simulink model "v13_aa_rii_16r128dr_ver104"  (Simulink 7.9)
settings: Solver=FixedStepDiscrete  SolverMode=SingleTasking  StartTime=0.0  StopTime=32768  RelTol=1e-3
undefined symbols (not defined in the file or in its folder): sfp
libraries: xbsIndex_r4 x19795, xps_library x54, casper_library_misc x36, dspsigops x32
(part 1 of 212)
settings Model: Creator=jayanth  LastModifiedBy=mwagner
settings DebuggingCC: FcnCallInpInsideContextMsg=Use local settings
settings GRTTargetCC: CombineOutputUpdateFcns=off  GRTInterface=on

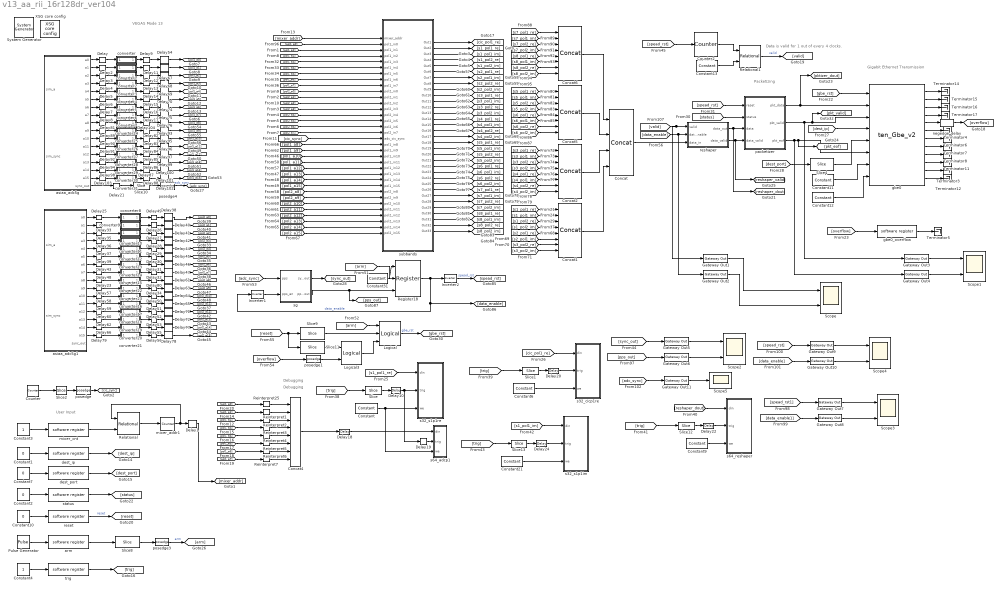
== system v13_aa_rii_16r128dr_ver104 ==
  note: Gigabit Ethernet Transmission
  note: Debugging
  note: Debugging
  note: Packetizing
  note: Data is valid for 1 out of every 4 clocks.
  note: VEGAS Mode 13
  note: User Input
  " System Generator" : xbsIndex_r4/ System Generator  Tag=genX  xilinxfamily=virtex6  part=xc6vsx475t  speed=-1  package=ff1759  synthesis_tool=XST  clock_wrapper=Clock Enables  directory=./sn/sysgen  proj_type=Project Navigator  Synth_file=XST Defaults  Impl_file=ISE Defaults  testbench=off  simulink_period=1  sysclk_period=5.3191  dcm_input_clock_period=100  incr_netlist=off  trim_vbits=Everywhere in SubSystem  dbl_ovrd=According to Block Masks  core_generation=According to Block Masks  run_coregen=off  deprecated_control=off  eval_field=0  block_type=sysgen
  Concat : xbsIndex_r4/Concat  num_inputs=4  dbl_ovrd=off  block_type=concat
  Concat{1..2,4..6} (5 alike) : xbsIndex_r4/Concat  num_inputs=8  dbl_ovrd=off  block_type=concat
  Constant{,6,9,21} (4 alike) : xbsIndex_r4/Constant  const=1  gui_display_data_type=Boolean  arith_type=Boolean  n_bits=16  bin_pt=14  preci_type=Single  exp_width=8  frac_width=24  explicit_period=off  period=1  equ=P=C  opselect=C  inp2=PCIN>>17  opr=+  inp1=P  carry=CIN  dbl_ovrd=off  block_type=constant
  Constant{1..2,7,10} (4 alike) : Constant  Value=0
  Constant11 : xbsIndex_r4/Constant  const=1  gui_display_data_type=Boolean  arith_type=Boolean  n_bits=16  bin_pt=14  preci_type=Single  exp_width=8  frac_width=24  explicit_period=on  period=1  equ=P=C  opselect=C  inp2=PCIN>>17  opr=+  inp1=P  carry=CIN  dbl_ovrd=off  block_type=constant
  Constant12 : xbsIndex_r4/Constant  const=0  gui_display_data_type=Boolean  arith_type=Boolean  n_bits=16  bin_pt=14  preci_type=Single  exp_width=8  frac_width=24  explicit_period=on  period=1  equ=P=C  opselect=C  inp2=PCIN>>17  opr=+  inp1=P  carry=CIN  dbl_ovrd=off  block_type=constant
  Constant13 : xbsIndex_r4/Constant  const=7  gui_display_data_type=Fixed-point  arith_type=Unsigned  n_bits=3  bin_pt=0  preci_type=Single  exp_width=8  frac_width=24  explicit_period=on  period=1  equ=P=C  opselect=C  inp2=PCIN>>17  opr=+  inp1=P  carry=CIN  dbl_ovrd=off  block_type=constant
  Constant3 : Constant
  Constant31 : xbsIndex_r4/Constant  const=1  gui_display_data_type=Boolean  arith_type=Boolean  n_bits=16  bin_pt=0  preci_type=Single  exp_width=8  frac_width=24  explicit_period=on  period=1  equ=P=C  opselect=C  inp2=PCIN>>17  opr=+  inp1=P  carry=CIN  dbl_ovrd=off  block_type=constant
      settings: block_version=11.4
  Constant4 : Constant
  Counter : xbsIndex_r4/Counter  cnt_type=Free Running  cnt_to=Inf  operation=Up  start_count=0  cnt_by_val=1  arith_type=Unsigned  n_bits=16  bin_pt=0  load_pin=off  rst=off  en=off  explicit_period=on  period=1  dbl_ovrd=off  use_behavioral_HDL=off  implementation=Fabric  block_type=counter
  Counter2 : xbsIndex_r4/Counter  cnt_type=Free Running  cnt_to=Inf  operation=Up  start_count=2  cnt_by_val=1  arith_type=Unsigned  n_bits=3  bin_pt=0  load_pin=off  rst=on  en=off  explicit_period=on  period=1  dbl_ovrd=off  use_behavioral_HDL=off  implementation=Fabric  block_type=counter
      settings: block_version=11.4
  Delay{,2..8,21,23,25,33,35..37,39,43,48,57..60,62,66,79,81,89..92,94,98,103} (33 alike) : xbsIndex_r4/Delay  rst=off  infoeditControl=Selection of Reset will increase slice count due to use of real FFs and instead of SRLs  en=off  latency=3  dbl_ovrd=off  reg_retiming=off  block_type=delay
  Delay1 : xbsIndex_r4/Delay  rst=off  infoeditControl=Selection of Reset will increase slice count due to use of real FFs and instead of SRLs  en=off  latency=2  dbl_ovrd=off  reg_retiming=on  block_type=delay
      settings: block_version=11.4
  Delay{10,18..20,22,24} (6 alike) : xbsIndex_r4/Delay  rst=off  infoeditControl=Selection of Reset will increase slice count due to use of real FFs and instead of SRLs  en=off  latency=20  dbl_ovrd=off  reg_retiming=off  block_type=delay
  Delay{38,40..42,44..47,54,61,63..65,67..72,74..78,93,95..97,99..102} (32 alike) : xbsIndex_r4/Delay  rst=off  infoeditControl=Selection of Reset will increase slice count due to use of real FFs and instead of SRLs  en=off  latency=3  dbl_ovrd=off  reg_retiming=on  block_type=delay
      settings: block_version=11.4
  Delay{9,11..17,26..32,34,49..53,55..56,80,82..88,104} (32 alike) : xbsIndex_r4/Delay  rst=off  infoeditControl=Selection of Reset will increase slice count due to use of real FFs and instead of SRLs  en=off  latency=8  dbl_ovrd=off  reg_retiming=off  block_type=delay
  Gateway Out{1..11} (11 alike) : xbsIndex_r4/Gateway Out  inherit_from_input=off  hdl_port=on  timing_constraint=None  locs_specified=off  LOCs={}  UseAsDAC=off  DACChannel='1'  block_type=gatewayout
      settings: infoedit="Gateway out block.  Converts Xilinx fixed point or floating-point type inputs into ouputs of type Simulink integer, double, or fixed point.<br><br>Hardware notes:  In hardware these blocks become top level output ports or are discarded, depending on how they are configured."
  Inverter1 : xbsIndex_r4/Inverter  en=off  latency=0  dbl_ovrd=off  block_type=inv
      settings: block_version=11.4
  Inverter2 : xbsIndex_r4/Inverter  en=off  latency=0  dbl_ovrd=off  block_type=inv
      settings: block_version=11.4
  Logical : xbsIndex_r4/Logical  logical_function=OR  inputs=3  en=off  latency=0  precision=Full  arith_type=Unsigned  n_bits=16  bin_pt=0  align_bp=on  dbl_ovrd=off  block_type=logical
  Logical3 : xbsIndex_r4/Logical  logical_function=AND  inputs=2  en=off  latency=0  precision=Full  arith_type=Unsigned  n_bits=8  bin_pt=2  align_bp=on  dbl_ovrd=off  block_type=logical
      settings: xl_area=[0, 0, 0, 0, 0, 0, 0]
  Pulse Generator : DiscretePulseGenerator  Period=10000  PhaseDelay=100
  Register18 : xbsIndex_r4/Register  init=0  rst=on  en=on  dbl_ovrd=off  block_type=register
      settings: block_version=11.4
  Reinterpret{1..7,25} (8 alike) : xbsIndex_r4/Reinterpret  force_arith_type=on  arith_type=Unsigned  force_bin_pt=on  bin_pt=0  block_type=reinterpret
  Relational : xbsIndex_r4/Relational  mode=a=b  en=off  latency=1  dbl_ovrd=off  block_type=relational
  Relational1 : xbsIndex_r4/Relational  mode=a=b  en=off  latency=0  dbl_ovrd=off  block_type=relational
  Scope{,1..4} (5 alike) : Scope  NumInputPorts=2
      Scope settings: YMin=-0.0075~-5  YMax=0.0075~5  SaveName=ScopeData4
      Scope1 settings: TimeRange=32768  YMin=-0.0075~-5  YMax=0.0075~5  SaveName=ScopeData5
      Scope2 settings: YMin=-0.0075~-5  YMax=0.0075~5  SaveName=ScopeData2
      Scope3 settings: YMin=-0.0075~-5  YMax=0.0075~5  SaveName=ScopeData6
      Scope4 settings: YMin=-0.0075~-5  YMax=0.0075~5  SaveName=ScopeData7
  Scope5 : Scope
      settings: YMin=-0.0075  YMax=0.0075  SaveName=ScopeData8
  Slice{,1,8..10,12..13} (7 alike) : xbsIndex_r4/Slice  nbits=1  boolean_output=on  mode=Lower Bit Location + Width  bit1=0  base1=MSB of Input  bit0=0  base0=LSB of Input  dbl_ovrd=off  block_type=slice
  Slice11 : xbsIndex_r4/Slice  nbits=1  boolean_output=on  mode=Lower Bit Location + Width  bit1=0  base1=MSB of Input  bit0=1  base0=LSB of Input  dbl_ovrd=off  block_type=slice
      settings: block_version=10.1.3
  Slice2 : xbsIndex_r4/Slice  nbits=1  boolean_output=on  mode=Upper Bit Location + Width  bit1=0  base1=MSB of Input  bit0=0  base0=LSB of Input  dbl_ovrd=off  block_type=slice
  Slice4 : xbsIndex_r4/Slice  nbits=16  boolean_output=off  mode=Lower Bit Location + Width  bit1=0  base1=MSB of Input  bit0=0  base0=LSB of Input  dbl_ovrd=off  block_type=slice
  Terminator{3..8,11..12,14..17} (12 alike) : Terminator
  XSG core config : xps_library/XSG core config  Tag=xps:xsg  hw_sys=ROACH2:sx475t  clk_src=adc0_clk  ROACH_clk_src=adc0_clk  ROACH2_clk_src=adc0_clk  clk_rate=188  sample_period=1  synthesis_tool=XST
  arm : xps_library/software register  Tag=xps:sw_reg  io_dir=From Processor  arith_type=Unsigned  bitwidth=32  bin_pt=0  sample_period=1  gw_name=v13_aa_rii_16r128dr_ver104_arm_user_data_out
      link override convert: arith_type=Unsigned  n_bits=32  bin_pt=0
      link override newmode13_arm_user_data_out: arith_type=Unsigned  n_bits=32  bin_pt=0  period=1
      link override r_spec_2048_r102_cnt_rst_user_data_out: arith_type=Unsigned  n_bits=32  bin_pt=0  period=1
      link override r_spec_2048_r102_nosim_cnt_rst_user_data_out: arith_type=Unsigned  n_bits=32  bin_pt=0  period=1
      link override r_spec_2048_r103_cnt_rst_user_data_out: arith_type=Unsigned  n_bits=32  bin_pt=0  period=1
      link override roach_spec_no10gbe_sync_cnt_rst_user_data_out: arith_type=Unsigned  n_bits=32  bin_pt=0  period=1
      link override roach_spec_rst_cnt_user_data_out: arith_type=Unsigned  n_bits=32  bin_pt=0  period=1
      link override roach_spec_rst_sync_cnt_user_data_out: arith_type=Unsigned  n_bits=32  bin_pt=0  period=1
  asiaa_adc5g : Subsystem  Tag=xps:adc5g  AncestorBlock=xps_library/ADCs/asiaa_adc5g  mask(adc5g){input_mode=One-channel -- A, adc_brd=ZDOK 0, demux=1:1, adc_bit_width=8, adc_clk_rate=600, using_ctrl=on, test_ramp=off}
      mask prompts: input_mode "Input mode:"; adc_brd "ADC Board"; demux "Internal demux"; adc_bit_width "ADC Resolution (bits):"; adc_clk_rate "ADC Clock Rate (MHz):"; using_ctrl "use control"; test_ramp "start in test ramp mode"
      mask help:
      | Interface for the ASIAA 5 GSps ADC. Supports interleaving all four
      | cores using either input A or C, or interleaving two-cores each for
      | A and C. The hardware itself comes in two versions:
      |
      | DMUX 1:2 -- 4-bit samples, 4 parallel streams
      | DMUX 1:1 -- 8-bit samples, 2 parallel streams
      |
      | In either configuration this block will output 8 parallel streams
      | but the underlying interface, and the bitwidth of the samples,
      | will be different.
      mask init:
      | adc5g_init(gcb,...
      |     'input_mode', input_mode,...
      |     'demux', demux,...
      |     'adc_clk_rate', adc_clk_rate,...
      |     'adc_bit_width', adc_bit_width,...
      |     'adc_brd', adc_brd,...
      |     'using_ctrl', using_ctrl,...
      |     'test_ramp', test_ramp);
      mask StyleString: popup(One-channel -- A|One-channel -- C|Two-channel -- A&C),popup(ZDOK 0|ZDOK 1),popup(1:1|1:2),edit,edit,checkbox,checkbox
      mask CallbackString:
      | ||demux = get_param(gcb,'demux');
      | if(strcmp(demux,'1:1')),
      |     set_param(gcb, 'adc_bit_width', '8')
      | elseif(strcmp(demux, '1:2')),
      |     set_param(gcb, 'adc_bit_width', '4');
      | else
      | end||||
  asiaa_adc5g1 : Subsystem  Tag=xps:adc5g  AncestorBlock=xps_library/ADCs/asiaa_adc5g  mask(adc5g){input_mode=One-channel -- A, adc_brd=ZDOK 1, demux=1:1, adc_bit_width=8, adc_clk_rate=600, using_ctrl=on, test_ramp=off}
      mask prompts: input_mode "Input mode:"; adc_brd "ADC Board"; demux "Internal demux"; adc_bit_width "ADC Resolution (bits):"; adc_clk_rate "ADC Clock Rate (MHz):"; using_ctrl "use control"; test_ramp "start in test ramp mode"
      mask help:
      | Interface for the ASIAA 5 GSps ADC. Supports interleaving all four
      | cores using either input A or C, or interleaving two-cores each for
      | A and C. The hardware itself comes in two versions:
      |
      | DMUX 1:2 -- 4-bit samples, 4 parallel streams
      | DMUX 1:1 -- 8-bit samples, 2 parallel streams
      |
      | In either configuration this block will output 8 parallel streams
      | but the underlying interface, and the bitwidth of the samples,
      | will be different.
      mask init: same as v13_aa_rii_16r128dr_ver104/asiaa_adc5g
      mask StyleString: popup(One-channel -- A|One-channel -- C|Two-channel -- A&C),popup(ZDOK 0|ZDOK 1),popup(1:1|1:2),edit,edit,checkbox,checkbox
      mask CallbackString:
      | ||demux = get_param(gcb,'demux');
      | if(strcmp(demux,'1:1')),
      |     set_param(gcb, 'adc_bit_width', '8')
      | elseif(strcmp(demux, '1:2')),
      |     set_param(gcb, 'adc_bit_width', '4');
      | else
      | end||||
  converter : Subsystem
  converter1 : Subsystem
  converter10 : Subsystem
  converter11 : Subsystem
  converter12 : Subsystem
  converter13 : Subsystem
  converter14 : Subsystem
  converter15 : Subsystem
  converter16 : Subsystem
  converter17 : Subsystem
  converter18 : Subsystem
  converter19 : Subsystem
  converter2 : Subsystem
  converter20 : Subsystem
  converter21 : Subsystem
  converter22 : Subsystem
  converter23 : Subsystem
  converter24 : Subsystem
  converter25 : Subsystem
  converter26 : Subsystem
  converter27 : Subsystem
  converter28 : Subsystem
  converter29 : Subsystem
  converter3 : Subsystem
  converter30 : Subsystem
  converter31 : Subsystem
  converter4 : Subsystem
  converter5 : Subsystem
  converter6 : Subsystem
  converter7 : Subsystem
  converter8 : Subsystem
  converter9 : Subsystem
  dest_ip : xps_library/software register  Tag=xps:sw_reg  io_dir=From Processor  arith_type=Unsigned  bitwidth=32  bin_pt=0  sample_period=1  gw_name=v13_aa_rii_16r128dr_ver104_dest_ip_user_data_out
      link override newmode13_dest_ip_user_data_out: arith_type=Unsigned  n_bits=32  bin_pt=0  period=1
      link override packetizer_dest_ip_user_data_out: arith_type=Unsigned  n_bits=32  bin_pt=0  period=1
  dest_port : xps_library/software register  Tag=xps:sw_reg  io_dir=From Processor  arith_type=Unsigned  bitwidth=32  bin_pt=0  sample_period=1  gw_name=v13_aa_rii_16r128dr_ver104_dest_port_user_data_out
      link override newmode13_dest_port_user_data_out: arith_type=Unsigned  n_bits=32  bin_pt=0  period=1
      link override packetizer_dest_port_user_data_out: arith_type=Unsigned  n_bits=32  bin_pt=0  period=1
  gbe0 : xps_library/ten_Gbe_v2  Tag=xps:tengbe_v2  flavour=sfp+  slot=0  port_r1=0  port_r2_cx4=0  port_r2_sfpp=0  rx_dist_ram=off  large_frames=on  show_param=on  pre_emph_r2=0.74  pre_emph=3  post_emph_r2=0.18  rxeqmix_r2=7  swing_r2=940  swing=800  fab_en=on  fab_mac=hex2dec('123456780000')  fab_ip=192*(2^24) + 168*(2^16) + 5*(2^8) + 20*(2^0)  fab_udp=10000  fab_gate=1  cpu_rx_en=on  cpu_tx_en=on
  gbe0_overflow : xps_library/software register  Tag=xps:sw_reg  io_dir=To Processor  arith_type=Unsigned  bitwidth=32  bin_pt=0  sample_period=1  gw_name=v13_aa_rii_16r128dr_ver104_gbe0_overflow_user_data_in
      link override convert: arith_type=Unsigned  n_bits=32  bin_pt=0
  mixer_addr1 : xbsIndex_r4/Counter  cnt_type=Free Running  cnt_to=Inf  operation=Up  start_count=0  cnt_by_val=1  arith_type=Unsigned  n_bits=11  bin_pt=0  load_pin=off  rst=on  en=off  explicit_period=on  period=1  dbl_ovrd=off  use_behavioral_HDL=off  implementation=Fabric  block_type=counter
      settings: block_version=11.4
  mixer_cnt : xps_library/software register  Tag=xps:sw_reg  io_dir=From Processor  arith_type=Unsigned  bitwidth=32  bin_pt=0  sample_period=1  gw_name=v13_aa_rii_16r128dr_ver104_mixer_cnt_user_data_out
      link override newmode13_mixer_cnt_user_data_out: arith_type=Unsigned  n_bits=32  bin_pt=0  period=1
  negedge_delay : casper_library_misc/negedge_delay  pulse_len=2^20
  packetizer : Subsystem  mask{header_words=2, data_words=1024}
      mask prompts: header_words "Number of 64-bit words in header"; data_words "Number of 64-bit words in data"
  posedge{,1,3..4} (4 alike) : casper_library_misc/posedge  Tag=Rising Edge Detector
  reset : xps_library/software register  Tag=xps:sw_reg  io_dir=From Processor  arith_type=Unsigned  bitwidth=32  bin_pt=0  sample_period=1  gw_name=v13_aa_rii_16r128dr_ver104_reset_user_data_out
      link override newmode13_reset_user_data_out: arith_type=Unsigned  n_bits=32  bin_pt=0  period=1
      link override packetizer_status_user_data_out: arith_type=Unsigned  n_bits=32  bin_pt=0  period=1
  reshaper : Subsystem
  s32_cicp1re : Subsystem  AncestorBlock=casper_library_scopes/snap  mask(snap){nsamples=16}
      mask prompts: nsamples "No. of samples(2^?)"
      mask help:
      | The <i>snap</i> block provides a packaged solution to capturing data from
      | the FPGA fabric and making it accessible from the CPU.  The block comes in
      | 2 flavors: <i>snap</i> (which captures to a 32 bit wide Shared BRAM), and
      | <i>snap64</i> (which captures to 2x32 bit wide Shared BRAMs to effect a
      | 64 bit capture).
      mask Help: eval('xlWeb(''http://casper.berkeley.edu/wiki/Snap'')')
  s32_s1p1im : Subsystem  AncestorBlock=casper_library_scopes/snap  mask(snap){nsamples=16}
      mask prompts: nsamples "No. of samples(2^?)"
      mask help:
      | The <i>snap</i> block provides a packaged solution to capturing data from
      | the FPGA fabric and making it accessible from the CPU.  The block comes in
      | 2 flavors: <i>snap</i> (which captures to a 32 bit wide Shared BRAM), and
      | <i>snap64</i> (which captures to 2x32 bit wide Shared BRAMs to effect a
      | 64 bit capture).
      mask Help: eval('xlWeb(''http://casper.berkeley.edu/wiki/Snap'')')
  s32_s1p1re : Subsystem  AncestorBlock=casper_library_scopes/snap  mask(snap){nsamples=16}
      mask prompts: nsamples "No. of samples(2^?)"
      mask help:
      | The <i>snap</i> block provides a packaged solution to capturing data from
      | the FPGA fabric and making it accessible from the CPU.  The block comes in
      | 2 flavors: <i>snap</i> (which captures to a 32 bit wide Shared BRAM), and
      | <i>snap64</i> (which captures to 2x32 bit wide Shared BRAMs to effect a
      | 64 bit capture).
      mask Help: eval('xlWeb(''http://casper.berkeley.edu/wiki/Snap'')')
  s64_adcp1 : Subsystem  AncestorBlock=casper_library_scopes/snap64  mask(snap64){nsamples=13}
      mask prompts: nsamples "No. of samples(2^?)"
      mask help:
      | The <i>snap</i> block provides a packaged solution to capturing data from
      | the FPGA fabric and making it accessible from the CPU.  The block comes in
      | 2 flavors: <i>snap</i> (which captures to a 32 bit wide Shared BRAM), and
      | <i>snap64</i> (which captures to 2x32 bit wide Shared BRAMs to effect a
      | 64 bit capture).
      mask Help: eval('xlWeb(''http://casper.berkeley.edu/wiki/Snap'')')
  s64_reshaper : Subsystem  AncestorBlock=casper_library_scopes/snap64  mask(snap64){nsamples=13}
      mask prompts: nsamples "No. of samples(2^?)"
      mask help:
      | The <i>snap</i> block provides a packaged solution to capturing data from
      | the FPGA fabric and making it accessible from the CPU.  The block comes in
      | 2 flavors: <i>snap</i> (which captures to a 32 bit wide Shared BRAM), and
      | <i>snap64</i> (which captures to 2x32 bit wide Shared BRAMs to effect a
      | 64 bit capture).
      mask Help: eval('xlWeb(''http://casper.berkeley.edu/wiki/Snap'')')
  sg : Subsystem
  status : xps_library/software register  Tag=xps:sw_reg  io_dir=From Processor  arith_type=Unsigned  bitwidth=32  bin_pt=0  sample_period=1  gw_name=v13_aa_rii_16r128dr_ver104_status_user_data_out
      link override newmode13_status_user_data_out: arith_type=Unsigned  n_bits=32  bin_pt=0  period=1
      link override packetizer_status_user_data_out: arith_type=Unsigned  n_bits=32  bin_pt=0  period=1
  subbands : Subsystem
  trig : xps_library/software register  Tag=xps:sw_reg  io_dir=From Processor  arith_type=Unsigned  bitwidth=32  bin_pt=0  sample_period=1  gw_name=v13_aa_rii_16r128dr_ver104_trig_user_data_out
      link override cic_filter_testing_to_compile_trig_user_data_out: arith_type=Unsigned  n_bits=32  bin_pt=0  period=1
      link override newmode13_trig_user_data_out: arith_type=Unsigned  n_bits=32  bin_pt=0  period=1
  wires:
  mixer_addr1/1 -> Delay1/1, Relational/1
  Delay1/1 -> [mixer_addr]
  Relational/1 -> mixer_addr1/1
  mixer_cnt/1 -> Relational/2
  Constant3/1 -> mixer_cnt/1
  Constant/1 -> s32_s1p1re/3(we), s64_adcp1/3(we)
  Slice/1 -> Delay10/1
  Delay10/1 -> s32_s1p1re/2(trig), Delay19/1
  Concat4/1 -> Delay18/1
  Reinterpret25/1 -> Concat4/1
  Reinterpret1/1 -> Concat4/2
  Reinterpret2/1 -> Concat4/3
  Reinterpret3/1 -> Concat4/4
  Reinterpret4/1 -> Concat4/5
  Reinterpret5/1 -> Concat4/6
  Reinterpret6/1 -> Concat4/7
  Reinterpret7/1 -> Concat4/8
  [pol1_a0] -> Reinterpret25/1
  [pol1_a1] -> Reinterpret1/1
  [pol1_a7] -> Reinterpret7/1
  [pol1_a6] -> Reinterpret6/1
  [pol1_a5] -> Reinterpret5/1
  [pol1_a4] -> Reinterpret4/1
  [pol1_a3] -> Reinterpret3/1
  [pol1_a2] -> Reinterpret2/1
  Delay54/1 -> [pol1_a0]
  Delay67/1 -> [pol1_a1]
  Delay68/1 -> [pol1_a2]
  Delay69/1 -> [pol1_a3]
  Delay70/1 -> [pol1_a4]
  Delay74/1 -> [pol1_a5]
  Delay75/1 -> [pol1_a6]
  Delay76/1 -> [pol1_a7]
  Delay/1 -> converter/1(u8_0)
  Delay2/1 -> converter1/1(u8_0)
  Delay3/1 -> converter2/1(u8_0)
  Delay4/1 -> converter3/1(u8_0)
  Delay5/1 -> converter4/1(u8_0)
  Delay6/1 -> converter5/1(u8_0)
  Delay7/1 -> converter6/1(u8_0)
  Delay8/1 -> converter7/1(u8_0)
  Delay9/1 -> Delay54/1
  Delay11/1 -> Delay67/1
  Delay12/1 -> Delay68/1
  Delay13/1 -> Delay69/1
  Delay14/1 -> Delay70/1
  Delay15/1 -> Delay74/1
  Delay16/1 -> Delay75/1
  Delay17/1 -> Delay76/1
  Counter/1 -> Slice2/1
  Slice2/1 -> posedge/1
  posedge/1 -> [cic_sync]
  Delay18/1 -> s64_adcp1/1(din)
  Delay19/1 -> s64_adcp1/2(trig)
  [s1_pol1_re] -> s32_s1p1re/1(din)
  gbe0_overflow/1 -> Terminator5/1
  Constant1/1 -> dest_ip/1
  Constant7/1 -> dest_port/1
  dest_ip/1 -> [dest_ip]
  dest_port/1 -> [dest_port]
  [dest_ip] -> gbe0/4
  [dest_port] -> Slice4/1
  Constant2/1 -> status/1
  status/1 -> [status]
  packetizer/3(pkt_eof) -> gbe0/6, [pkt_eof], Gateway Out4/1
  [overflow] -> gbe0_overflow/1
  [spead_rst] -> packetizer/1(reset)
  [status] -> packetizer/2(status)
  subbands/2(Out2) -> [s1_pol1_re]
  subbands/3(Out3) -> [s1_pol1_im]
  subbands/4(Out4) -> [s1_pol2_re]
  subbands/5(Out5) -> [s1_pol2_im]
  reset/1 -> [reset]  "reset"
  Slice4/1 -> gbe0/5
  gbe0/6 -> Terminator4/1
  gbe0/7 -> Terminator6/1
  gbe0/8 -> Terminator7/1
  gbe0/9 -> Terminator8/1
  gbe0/10 -> Terminator11/1
  gbe0/11 -> Terminator3/1
  Constant11/1 -> gbe0/7
  gbe0/1 -> Terminator14/1
  gbe0/2 -> Terminator15/1
  gbe0/3 -> Terminator16/1
  gbe0/4 -> Terminator17/1
  gbe0/5 -> negedge_delay/1
  Constant12/1 -> gbe0/8
  gbe0/12 -> Terminator12/1
  [gbe_rst] -> gbe0/1
  negedge_delay/1 -> [overflow]
  Constant13/1 -> Relational1/2
  Counter2/1 -> Relational1/1
  Relational1/1 -> [valid]  "valid"
  Constant10/1 -> reset/1
  Constant31/1 -> Register18/1
  sg/2(pps_out) -> Register18/3, [pps_out]
  Register18/1 -> Inverter2/1, Inverter1/1, [data_enable]  "data_enable"
  arm/1 -> Slice8/1
  Pulse Generator/1 -> arm/1
  Inverter1/1 -> sg/2(pps_en)
  posedge1/1 -> Logical3/2
  Slice11/1 -> Logical3/1
  Slice9/1 -> Logical/2
  Logical3/1 -> Logical/3
  [arm] -> Register18/2
  [adc_sync] -> sg/1(pps)
  sg/1(sync_out) -> [sync_out]
  Inverter2/1 -> [spead_rst]  "spead_rst"
  Logical/1 -> [gbe_rst]  "gbe_rst"
  [overflow] -> posedge1/1
  [reset] -> Slice9/1, Slice11/1
  Delay21/1 -> Slice10/1
  Slice10/1 -> posedge4/1
  posedge4/1 -> [adc_sync]  "adc_sync"
  [spead_rst] -> Counter2/1
  Slice8/1 -> posedge3/1
  posedge3/1 -> [arm]  "arm"
  [arm] -> Logical/1
  [valid] -> reshaper/1(valid), Gateway Out1/1
  [data_enable] -> reshaper/2(data_enable), Gateway Out2/1
  reshaper/1(data_out) -> packetizer/3(data), [reshaper_dout]
  reshaper/2(data_valid) -> [reshaper_valid], packetizer/4(data_valid)
  Constant6/1 -> s32_cicp1re/3(we)
  Slice1/1 -> Delay20/1
  Delay20/1 -> s32_cicp1re/2(trig)
  [cic_pol1_re] -> s32_cicp1re/1(din)
  Constant4/1 -> trig/1
  trig/1 -> [trig]
  [trig] -> Slice/1
  [trig] -> Slice1/1
  Constant9/1 -> s64_reshaper/3(we)
  [reshaper_dout] -> s64_reshaper/1(din)
  Slice12/1 -> Delay22/1
  [trig] -> Slice12/1
  Delay22/1 -> s64_reshaper/2(trig)
  packetizer/1(pkt_data) -> gbe0/2, [pktizer_dout]
  packetizer/2(pkt_valid) -> gbe0/3, [pkt_valid], Gateway Out3/1
  [s1_pol1_re] -> Concat1/1
  [s1_pol1_im] -> Concat1/2
  [s1_pol2_re] -> Concat1/3
  [s1_pol2_im] -> Concat1/4
  Concat6/1 -> Concat/1
  Concat5/1 -> Concat/2
  Concat2/1 -> Concat/3
  Concat1/1 -> Concat/4
  Concat/1 -> reshaper/3(data_in)
  Constant21/1 -> s32_s1p1im/3(we)
  Slice13/1 -> Delay24/1
  Delay24/1 -> s32_s1p1im/2(trig)
  [s1_pol1_im] -> s32_s1p1im/1(din)
  [trig] -> Slice13/1
  Delay38/1 -> [pol2_a0]
  Delay40/1 -> [pol2_a1]
  Delay41/1 -> [pol2_a2]
  Delay42/1 -> [pol2_a3]
  Delay44/1 -> [pol2_a4]
  Delay45/1 -> [pol2_a5]
  Delay46/1 -> [pol2_a6]
  Delay47/1 -> [pol2_a7]
  Delay25/1 -> converter8/1(u8_0)
  Delay33/1 -> converter9/1(u8_0)
  Delay35/1 -> converter10/1(u8_0)
  Delay36/1 -> converter11/1(u8_0)
  Delay37/1 -> converter12/1(u8_0)
  Delay39/1 -> converter13/1(u8_0)
  Delay43/1 -> converter14/1(u8_0)
  Delay48/1 -> converter15/1(u8_0)
  Delay49/1 -> Delay38/1
  Delay26/1 -> Delay40/1
  Delay27/1 -> Delay41/1
  Delay28/1 -> Delay42/1
  Delay29/1 -> Delay44/1
  Delay30/1 -> Delay45/1
  Delay31/1 -> Delay46/1
  Delay32/1 -> Delay47/1
  converter/1(s8_7) -> Delay9/1
  converter1/1(s8_7) -> Delay11/1
  converter2/1(s8_7) -> Delay12/1
  converter3/1(s8_7) -> Delay13/1
  converter4/1(s8_7) -> Delay14/1
  converter5/1(s8_7) -> Delay15/1
  converter6/1(s8_7) -> Delay16/1
  converter7/1(s8_7) -> Delay17/1
  converter8/1(s8_7) -> Delay49/1
  converter9/1(s8_7) -> Delay26/1
  converter10/1(s8_7) -> Delay27/1
  converter11/1(s8_7) -> Delay28/1
  converter12/1(s8_7) -> Delay29/1
  converter13/1(s8_7) -> Delay30/1
  converter14/1(s8_7) -> Delay31/1
  converter15/1(s8_7) -> Delay32/1
  Delay61/1 -> [pol2_a8]
  Delay63/1 -> [pol2_a9]
  Delay64/1 -> [pol2_a10]
  Delay65/1 -> [pol2_a11]
  Delay71/1 -> [pol2_a12]
  Delay72/1 -> [pol2_a13]
  Delay77/1 -> [pol2_a14]
  Delay78/1 -> [pol2_a15]
  Delay23/1 -> converter22/1(u8_0)
  Delay57/1 -> converter23/1(u8_0)
  Delay58/1 -> converter16/1(u8_0)
  Delay59/1 -> converter17/1(u8_0)
  Delay60/1 -> converter18/1(u8_0)
  Delay62/1 -> converter19/1(u8_0)
  Delay66/1 -> converter20/1(u8_0)
  Delay79/1 -> converter21/1(u8_0)
  Delay80/1 -> Delay61/1
  Delay34/1 -> Delay63/1
  Delay50/1 -> Delay64/1
  Delay51/1 -> Delay65/1
  Delay52/1 -> Delay71/1
  Delay53/1 -> Delay72/1
  Delay55/1 -> Delay77/1
  Delay56/1 -> Delay78/1
  converter22/1(s8_7) -> Delay80/1
  converter23/1(s8_7) -> Delay34/1
  converter16/1(s8_7) -> Delay50/1
  converter17/1(s8_7) -> Delay51/1
  converter18/1(s8_7) -> Delay52/1
  converter19/1(s8_7) -> Delay53/1
  converter20/1(s8_7) -> Delay55/1
  converter21/1(s8_7) -> Delay56/1
  asiaa_adc5g1/1(a0) -> Delay25/1
  asiaa_adc5g1/2(a1) -> Delay33/1
  asiaa_adc5g1/3(a2) -> Delay35/1
  asiaa_adc5g1/4(a3) -> Delay36/1
  asiaa_adc5g1/5(a4) -> Delay37/1
  asiaa_adc5g1/6(a5) -> Delay39/1
  asiaa_adc5g1/7(a6) -> Delay43/1
  asiaa_adc5g1/8(a7) -> Delay48/1
  asiaa_adc5g1/9(a8) -> Delay23/1
  asiaa_adc5g1/10(a9) -> Delay57/1
  asiaa_adc5g1/11(a10) -> Delay58/1
  asiaa_adc5g1/12(a11) -> Delay59/1
  asiaa_adc5g1/13(a12) -> Delay60/1
  asiaa_adc5g1/14(a13) -> Delay62/1
  asiaa_adc5g1/15(a14) -> Delay66/1
  asiaa_adc5g1/16(a15) -> Delay79/1
  asiaa_adc5g/17(sync_out) -> Delay21/1
  Delay93/1 -> [pol1_a8]
  Delay95/1 -> [pol1_a9]
  Delay96/1 -> [pol1_a10]
  Delay97/1 -> [pol1_a11]
  Delay99/1 -> [pol1_a12]
  Delay100/1 -> [pol1_a13]
  Delay101/1 -> [pol1_a14]
  Delay102/1 -> [pol1_a15]
  Delay81/1 -> converter24/1(u8_0)
  Delay89/1 -> converter25/1(u8_0)
  Delay90/1 -> converter26/1(u8_0)
  Delay91/1 -> converter27/1(u8_0)
  Delay92/1 -> converter28/1(u8_0)
  Delay94/1 -> converter29/1(u8_0)
  Delay98/1 -> converter30/1(u8_0)
  Delay103/1 -> converter31/1(u8_0)
  Delay104/1 -> Delay93/1
  Delay82/1 -> Delay95/1
  Delay83/1 -> Delay96/1
  Delay84/1 -> Delay97/1
  Delay85/1 -> Delay99/1
  Delay86/1 -> Delay100/1
  Delay87/1 -> Delay101/1
  Delay88/1 -> Delay102/1
  converter24/1(s8_7) -> Delay104/1
  converter25/1(s8_7) -> Delay82/1
  converter26/1(s8_7) -> Delay83/1
  converter27/1(s8_7) -> Delay84/1
  converter28/1(s8_7) -> Delay85/1
  converter29/1(s8_7) -> Delay86/1
  converter30/1(s8_7) -> Delay87/1
  converter31/1(s8_7) -> Delay88/1
  asiaa_adc5g/1(a0) -> Delay/1
  asiaa_adc5g/2(a1) -> Delay2/1
  asiaa_adc5g/3(a2) -> Delay3/1
  asiaa_adc5g/4(a3) -> Delay4/1
  asiaa_adc5g/5(a4) -> Delay5/1
  asiaa_adc5g/6(a5) -> Delay6/1
  asiaa_adc5g/7(a6) -> Delay7/1
  asiaa_adc5g/8(a7) -> Delay8/1
  asiaa_adc5g/9(a8) -> Delay81/1
  asiaa_adc5g/10(a9) -> Delay89/1
  asiaa_adc5g/11(a10) -> Delay90/1
  asiaa_adc5g/12(a11) -> Delay91/1
  asiaa_adc5g/13(a12) -> Delay92/1
  asiaa_adc5g/14(a13) -> Delay94/1
  asiaa_adc5g/15(a14) -> Delay98/1
  asiaa_adc5g/16(a15) -> Delay103/1
  subbands/1(Out1) -> [cic_pol1_re]
  subbands/6(Out6) -> [s2_pol1_re]
  subbands/7(Out7) -> [s2_pol1_im]
  subbands/8(Out8) -> [s2_pol2_re]
  subbands/9(Out9) -> [s2_pol2_im]
  subbands/10(Out10) -> [s3_pol1_re]
  subbands/11(Out11) -> [s3_pol1_im]
  subbands/12(Out12) -> [s3_pol2_re]
  subbands/13(Out13) -> [s3_pol2_im]
  subbands/14(Out14) -> [s4_pol1_re]
  subbands/15(Out15) -> [s4_pol1_im]
  subbands/16(Out16) -> [s4_pol2_re]
  subbands/17(Out17) -> [s4_pol2_im]
  subbands/18(Out18) -> [s5_pol1_re]
  subbands/19(Out19) -> [s5_pol1_im]
  subbands/20(Out20) -> [s5_pol2_re]
  subbands/21(Out21) -> [s5_pol2_im]
  subbands/22(Out22) -> [s6_pol1_re]
  subbands/23(Out23) -> [s6_pol1_im]
  subbands/24(Out24) -> [s6_pol2_re]
  subbands/25(Out25) -> [s6_pol2_im]
  subbands/26(Out26) -> [s7_pol1_re]
  subbands/27(Out27) -> [s7_pol1_im]
  subbands/28(Out28) -> [s7_pol2_re]
  subbands/29(Out29) -> [s7_pol2_im]
  subbands/30(Out30) -> [s8_pol1_re]
  subbands/31(Out31) -> [s8_pol1_im]
  subbands/32(Out32) -> [s8_pol2_re]
  subbands/33(Out33) -> [s8_pol2_im]
  [s2_pol1_re] -> Concat1/5
  [s2_pol1_im] -> Concat1/6
  [s3_pol2_re] -> Concat1/7
  [s3_pol2_im] -> Concat1/8
  [s7_pol1_re] -> Concat6/1
  [s5_pol1_re] -> Concat5/1
  [s3_pol1_re] -> Concat2/1
  [s7_pol1_im] -> Concat6/2
  [s7_pol2_re] -> Concat6/3
  [s7_pol2_im] -> Concat6/4
  [s8_pol1_re] -> Concat6/5
  [s8_pol1_im] -> Concat6/6
  [s8_pol2_re] -> Concat6/7
  [s8_pol2_im] -> Concat6/8
  [s5_pol1_im] -> Concat5/2
  [s5_pol2_re] -> Concat5/3
  [s5_pol2_im] -> Concat5/4
  [s6_pol1_re] -> Concat5/5
  [s6_pol1_im] -> Concat5/6
  [s6_pol2_re] -> Concat5/7
  [s6_pol2_im] -> Concat5/8
  [s3_pol1_im] -> Concat2/2
  [s3_pol2_re] -> Concat2/3
  [s3_pol2_im] -> Concat2/4
  [s4_pol1_re] -> Concat2/5
  [s4_pol1_im] -> Concat2/6
  [s4_pol2_re] -> Concat2/7
  [s4_pol2_im] -> Concat2/8
  Gateway Out1/1 -> Scope/1
  Gateway Out2/1 -> Scope/2
  Gateway Out3/1 -> Scope1/1
  Gateway Out4/1 -> Scope1/2
  [mixer_addr] -> subbands/1(mixer_addr)
  [cic_sync] -> subbands/18(adc_cic_sync)
  [pol1_a0] -> subbands/2(pol1_in0)
  [pol1_a1] -> subbands/3(pol1_in1)
  [pol1_a2] -> subbands/4(pol1_in2)
  [pol1_a3] -> subbands/5(pol1_in3)
  [pol1_a4] -> subbands/6(pol1_in4)
  [pol1_a5] -> subbands/7(pol1_in5)
  [pol1_a6] -> subbands/8(pol1_in6)
  [pol1_a7] -> subbands/9(pol1_in7)
  [pol2_a0] -> subbands/10(pol2_in0)
  [pol2_a1] -> subbands/11(pol2_in1)
  [pol2_a2] -> subbands/12(pol2_In2)
  [pol2_a3] -> subbands/13(pol2_in3)
  [pol2_a4] -> subbands/14(pol2_in4)
  [pol2_a5] -> subbands/15(pol2_in5)
  [pol2_a6] -> subbands/16(pol2_in6)
  [pol2_a7] -> subbands/17(pol2_in7)
  [pol1_a8] -> subbands/19(pol1_in8)
  [pol1_a9] -> subbands/20(pol1_in9)
  [pol1_a10] -> subbands/21(pol1_in10)
  [pol1_a11] -> subbands/22(pol1_in11)
  [pol1_a12] -> subbands/23(pol1_in12)
  [pol1_a13] -> subbands/24(pol1_in13)
  [pol1_a14] -> subbands/25(pol1_in14)
  [pol1_a15] -> subbands/26(pol1_in15)
  [pol2_a8] -> subbands/27(pol2_in8)
  [pol2_a9] -> subbands/28(pol2_in9)
  [pol2_a10] -> subbands/29(pol2_in10)
  [pol2_a11] -> subbands/30(pol2_in11)
  [pol2_a12] -> subbands/31(pol2_in12)
  [pol2_a13] -> subbands/32(pol2_in13)
  [pol2_a14] -> subbands/33(pol2_in14)
  [pol2_a15] -> subbands/34(pol2_in15)
  Gateway Out5/1 -> Scope2/1
  Gateway Out6/1 -> Scope2/2
  [sync_out] -> Gateway Out5/1
  [pps_out] -> Gateway Out6/1
  Gateway Out7/1 -> Scope3/1
  Gateway Out8/1 -> Scope3/2
  [spead_rst1] -> Gateway Out7/1
  [data_enable1] -> Gateway Out8/1
  Gateway Out9/1 -> Scope4/1
  Gateway Out10/1 -> Scope4/2
  [spead_rst] -> Gateway Out9/1
  [data_enable] -> Gateway Out10/1
  Gateway Out11/1 -> Scope5/1
  [adc_sync] -> Gateway Out11/1

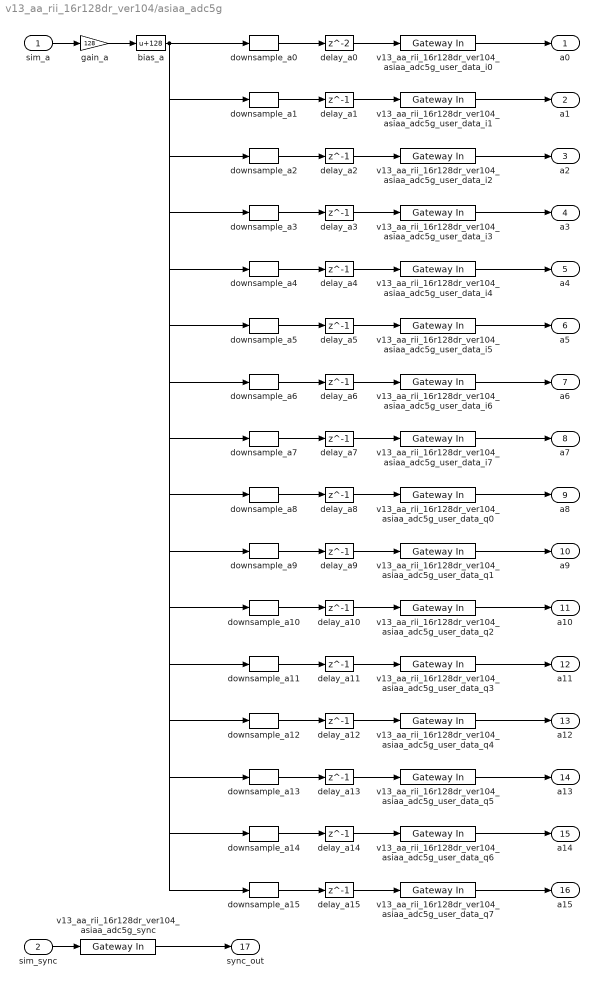
== system v13_aa_rii_16r128dr_ver104/asiaa_adc5g ==
  sim_a : Inport
  sim_sync : Inport  Port=2
  bias_a : Bias  Bias=128  SaturateOnIntegerOverflow=on
  delay_a0 : Delay  InitialCondition=0
  delay_a{1..15} (15 alike) : Delay  DelayLength=1  InitialCondition=0
  downsample_a0 : dspsigops/Downsample  N=16  phase=0  InputProcessing=Columns as channels (frame based)  RateOptions=Enforce single-rate processing  smode=Allow multirate  fmode=Maintain input frame size  ic=0
  downsample_a1 : dspsigops/Downsample  N=16  phase=1  InputProcessing=Columns as channels (frame based)  RateOptions=Enforce single-rate processing  smode=Allow multirate  fmode=Maintain input frame size  ic=0
  downsample_a10 : dspsigops/Downsample  N=16  phase=10  InputProcessing=Columns as channels (frame based)  RateOptions=Enforce single-rate processing  smode=Allow multirate  fmode=Maintain input frame size  ic=0
  downsample_a11 : dspsigops/Downsample  N=16  phase=11  InputProcessing=Columns as channels (frame based)  RateOptions=Enforce single-rate processing  smode=Allow multirate  fmode=Maintain input frame size  ic=0
  downsample_a12 : dspsigops/Downsample  N=16  phase=12  InputProcessing=Columns as channels (frame based)  RateOptions=Enforce single-rate processing  smode=Allow multirate  fmode=Maintain input frame size  ic=0
  downsample_a13 : dspsigops/Downsample  N=16  phase=13  InputProcessing=Columns as channels (frame based)  RateOptions=Enforce single-rate processing  smode=Allow multirate  fmode=Maintain input frame size  ic=0
  downsample_a14 : dspsigops/Downsample  N=16  phase=14  InputProcessing=Columns as channels (frame based)  RateOptions=Enforce single-rate processing  smode=Allow multirate  fmode=Maintain input frame size  ic=0
  downsample_a15 : dspsigops/Downsample  N=16  phase=15  InputProcessing=Columns as channels (frame based)  RateOptions=Enforce single-rate processing  smode=Allow multirate  fmode=Maintain input frame size  ic=0
  downsample_a2 : dspsigops/Downsample  N=16  phase=2  InputProcessing=Columns as channels (frame based)  RateOptions=Enforce single-rate processing  smode=Allow multirate  fmode=Maintain input frame size  ic=0
  downsample_a3 : dspsigops/Downsample  N=16  phase=3  InputProcessing=Columns as channels (frame based)  RateOptions=Enforce single-rate processing  smode=Allow multirate  fmode=Maintain input frame size  ic=0
  downsample_a4 : dspsigops/Downsample  N=16  phase=4  InputProcessing=Columns as channels (frame based)  RateOptions=Enforce single-rate processing  smode=Allow multirate  fmode=Maintain input frame size  ic=0
  downsample_a5 : dspsigops/Downsample  N=16  phase=5  InputProcessing=Columns as channels (frame based)  RateOptions=Enforce single-rate processing  smode=Allow multirate  fmode=Maintain input frame size  ic=0
  downsample_a6 : dspsigops/Downsample  N=16  phase=6  InputProcessing=Columns as channels (frame based)  RateOptions=Enforce single-rate processing  smode=Allow multirate  fmode=Maintain input frame size  ic=0
  downsample_a7 : dspsigops/Downsample  N=16  phase=7  InputProcessing=Columns as channels (frame based)  RateOptions=Enforce single-rate processing  smode=Allow multirate  fmode=Maintain input frame size  ic=0
  downsample_a8 : dspsigops/Downsample  N=16  phase=8  InputProcessing=Columns as channels (frame based)  RateOptions=Enforce single-rate processing  smode=Allow multirate  fmode=Maintain input frame size  ic=0
  downsample_a9 : dspsigops/Downsample  N=16  phase=9  InputProcessing=Columns as channels (frame based)  RateOptions=Enforce single-rate processing  smode=Allow multirate  fmode=Maintain input frame size  ic=0
  gain_a : Gain  Gain=128  SaturateOnIntegerOverflow=on  OutDataTypeStr=Inherit: Same as input  ParamDataTypeStr=Inherit: Same as input
  v13_aa_rii_16r128dr_ver104_asiaa_adc5g_sync : xbsIndex_r4/Gateway In  gui_display_data_type=Fixed-point  arith_type=Boolean  n_bits=16  bin_pt=14  preci_type=Single  exp_width=8  frac_width=24  quantization="Round  (unbiased: +/- Inf)"  overflow=Saturate  period=1  dbl_ovrd=off  timing_constraint=None  locs_specified=off  LOCs={}  UseAsADC=off  ADCChannel='1'  inherit_from_input=off  hdl_port=on  block_type=gatewayin
      settings: infoedit="Gateway in block.  Converts inputs of type Simulink integer, double and fixed point to  Xilinx fixed point or floating-point data type.<br><br>Hardware notes:  In hardware these blocks become top level input ports."
  v13_aa_rii_16r128dr_ver104_asiaa_adc5g_user_data_i{0..7} (8 alike) : xbsIndex_r4/Gateway In  gui_display_data_type=Fixed-point  arith_type=Unsigned  n_bits=8  bin_pt=0  preci_type=Single  exp_width=8  frac_width=24  quantization=Truncate  overflow=Wrap  period=1  dbl_ovrd=off  timing_constraint=None  locs_specified=off  LOCs={}  UseAsADC=off  ADCChannel='1'  inherit_from_input=off  hdl_port=on  block_type=gatewayin
      settings: infoedit="Gateway in block.  Converts inputs of type Simulink integer, double and fixed point to  Xilinx fixed point or floating-point data type.<br><br>Hardware notes:  In hardware these blocks become top level input ports."
  v13_aa_rii_16r128dr_ver104_asiaa_adc5g_user_data_q{0..7} (8 alike) : xbsIndex_r4/Gateway In  gui_display_data_type=Fixed-point  arith_type=Unsigned  n_bits=8  bin_pt=0  preci_type=Single  exp_width=8  frac_width=24  quantization=Truncate  overflow=Wrap  period=1  dbl_ovrd=off  timing_constraint=None  locs_specified=off  LOCs={}  UseAsADC=off  ADCChannel='1'  inherit_from_input=off  hdl_port=on  block_type=gatewayin
      settings: infoedit="Gateway in block.  Converts inputs of type Simulink integer, double and fixed point to  Xilinx fixed point or floating-point data type.<br><br>Hardware notes:  In hardware these blocks become top level input ports."
  a0 : Outport
  a1 : Outport  Port=2
  a2 : Outport  Port=3
  a3 : Outport  Port=4
  a4 : Outport  Port=5
  a5 : Outport  Port=6
  a6 : Outport  Port=7
  a7 : Outport  Port=8
  a8 : Outport  Port=9
  a9 : Outport  Port=10
  a10 : Outport  Port=11
  a11 : Outport  Port=12
  a12 : Outport  Port=13
  a13 : Outport  Port=14
  a14 : Outport  Port=15
  a15 : Outport  Port=16
  sync_out : Outport  Port=17
  wires:
  sim_a/1 -> gain_a/1
  gain_a/1 -> bias_a/1
  bias_a/1 -> downsample_a0/1, downsample_a1/1, downsample_a2/1, downsample_a3/1, downsample_a4/1, downsample_a5/1, downsample_a6/1, downsample_a7/1, downsample_a8/1, downsample_a9/1, downsample_a10/1, downsample_a11/1, downsample_a12/1, downsample_a13/1, downsample_a14/1, downsample_a15/1
  downsample_a0/1 -> delay_a0/1
  delay_a0/1 -> v13_aa_rii_16r128dr_ver104_asiaa_adc5g_user_data_i0/1
  v13_aa_rii_16r128dr_ver104_asiaa_adc5g_user_data_i0/1 -> a0/1
  downsample_a1/1 -> delay_a1/1
  delay_a1/1 -> v13_aa_rii_16r128dr_ver104_asiaa_adc5g_user_data_i1/1
  v13_aa_rii_16r128dr_ver104_asiaa_adc5g_user_data_i1/1 -> a1/1
  downsample_a2/1 -> delay_a2/1
  delay_a2/1 -> v13_aa_rii_16r128dr_ver104_asiaa_adc5g_user_data_i2/1
  v13_aa_rii_16r128dr_ver104_asiaa_adc5g_user_data_i2/1 -> a2/1
  downsample_a3/1 -> delay_a3/1
  delay_a3/1 -> v13_aa_rii_16r128dr_ver104_asiaa_adc5g_user_data_i3/1
  v13_aa_rii_16r128dr_ver104_asiaa_adc5g_user_data_i3/1 -> a3/1
  downsample_a4/1 -> delay_a4/1
  delay_a4/1 -> v13_aa_rii_16r128dr_ver104_asiaa_adc5g_user_data_i4/1
  v13_aa_rii_16r128dr_ver104_asiaa_adc5g_user_data_i4/1 -> a4/1
  downsample_a5/1 -> delay_a5/1
  delay_a5/1 -> v13_aa_rii_16r128dr_ver104_asiaa_adc5g_user_data_i5/1
  v13_aa_rii_16r128dr_ver104_asiaa_adc5g_user_data_i5/1 -> a5/1
  downsample_a6/1 -> delay_a6/1
  delay_a6/1 -> v13_aa_rii_16r128dr_ver104_asiaa_adc5g_user_data_i6/1
  v13_aa_rii_16r128dr_ver104_asiaa_adc5g_user_data_i6/1 -> a6/1
  downsample_a7/1 -> delay_a7/1
  delay_a7/1 -> v13_aa_rii_16r128dr_ver104_asiaa_adc5g_user_data_i7/1
  v13_aa_rii_16r128dr_ver104_asiaa_adc5g_user_data_i7/1 -> a7/1
  downsample_a8/1 -> delay_a8/1
  delay_a8/1 -> v13_aa_rii_16r128dr_ver104_asiaa_adc5g_user_data_q0/1
  v13_aa_rii_16r128dr_ver104_asiaa_adc5g_user_data_q0/1 -> a8/1
  downsample_a9/1 -> delay_a9/1
  delay_a9/1 -> v13_aa_rii_16r128dr_ver104_asiaa_adc5g_user_data_q1/1
  v13_aa_rii_16r128dr_ver104_asiaa_adc5g_user_data_q1/1 -> a9/1
  downsample_a10/1 -> delay_a10/1
  delay_a10/1 -> v13_aa_rii_16r128dr_ver104_asiaa_adc5g_user_data_q2/1
  v13_aa_rii_16r128dr_ver104_asiaa_adc5g_user_data_q2/1 -> a10/1
  downsample_a11/1 -> delay_a11/1
  delay_a11/1 -> v13_aa_rii_16r128dr_ver104_asiaa_adc5g_user_data_q3/1
  v13_aa_rii_16r128dr_ver104_asiaa_adc5g_user_data_q3/1 -> a11/1
  downsample_a12/1 -> delay_a12/1
  delay_a12/1 -> v13_aa_rii_16r128dr_ver104_asiaa_adc5g_user_data_q4/1
  v13_aa_rii_16r128dr_ver104_asiaa_adc5g_user_data_q4/1 -> a12/1
  downsample_a13/1 -> delay_a13/1
  delay_a13/1 -> v13_aa_rii_16r128dr_ver104_asiaa_adc5g_user_data_q5/1
  v13_aa_rii_16r128dr_ver104_asiaa_adc5g_user_data_q5/1 -> a13/1
  downsample_a14/1 -> delay_a14/1
  delay_a14/1 -> v13_aa_rii_16r128dr_ver104_asiaa_adc5g_user_data_q6/1
  v13_aa_rii_16r128dr_ver104_asiaa_adc5g_user_data_q6/1 -> a14/1
  downsample_a15/1 -> delay_a15/1
  delay_a15/1 -> v13_aa_rii_16r128dr_ver104_asiaa_adc5g_user_data_q7/1
  v13_aa_rii_16r128dr_ver104_asiaa_adc5g_user_data_q7/1 -> a15/1
  sim_sync/1 -> v13_aa_rii_16r128dr_ver104_asiaa_adc5g_sync/1
  v13_aa_rii_16r128dr_ver104_asiaa_adc5g_sync/1 -> sync_out/1
